simulink model "s_c856m1kfloat_sim"  (R2016b) (continued)
== system s_c856m1kfloat_sim == (continued)
      mask prompts: io_dir "I/O direction"; io_delay "I/O delay"; init_val "Initial Value"; sample_period "Sample period"; names "Bitfield names [msb...lsb]"; bitwidths "Bitfield widths"; bin_pts "Bitfield binary pts"; arith_types "Bitfield types, ufix=0, fix=1, bool=2"; sim_port "Provide sim input/output?"; show_format "Print format string?"
      mask help: A 32-bit software-accessible register. Can be divided into bitfields of varying widths and types using the fields in the block mask.
      mask init:
      | swreg_init(gcb)
      settings: AttributesFormatString=1output:uf32.0 = 32 bits
      mask TypeOptions io_dir: To Processor | From Processor
      mask Callback io_dir:
      | mask_enables = get_param(gcb, 'MaskEnables');
      | mask_names = get_param(gcb, 'MaskNames');
      | if strcmp(get_param(gcb, 'io_dir'), 'To Processor'),
      |     mask_enables{ismember(mask_names, 'sample_period')} = 'off';
      | else
      |     mask_enables{ismember(mask_names, 'sample_period')} = 'on';
      | end
      | set_param(gcb, 'MaskEnables', mask_enables);
      mask DialogControl ParameterTabVar0 Prompt: Setup
  wb_prog_delay : Subsystem  mask{n_bits_fft=fft_stages, n_bits_cd=17}
      mask prompts: n_bits_fft "fft length (2^?)"; n_bits_cd "max coarse delay (2^?)"
  wb_prog_delay1 : Subsystem  mask{n_bits_fft=fft_stages, n_bits_cd=17}
      mask prompts: n_bits_fft "fft length (2^?)"; n_bits_cd "max coarse delay (2^?)"
  we_delay112 : Subsystem  AncestorBlock=casper_library_delays/pipeline  mask(pipeline){latency=2}
      mask help:
      | An explicitly laid-out delay line to avoid mapping to SRL16s
      | for use in pipelining to help achieve timing closure.
      mask init:
      | pipeline_init(gcb,'latency',latency);
      settings: AttributesFormatString=Latency = %<latency>
      mask Display:
      | port_label('input', 1, 'd');
      | port_label('output', 1, 'q');
      | disp(['Z^-', num2str(latency)]);
  we_delay113 : Subsystem  AncestorBlock=casper_library_delays/pipeline  mask(pipeline){latency=2}
      mask help:
      | An explicitly laid-out delay line to avoid mapping to SRL16s
      | for use in pipelining to help achieve timing closure.
      mask init:
      | pipeline_init(gcb,'latency',latency);
      settings: AttributesFormatString=Latency = %<latency>
      mask Display:
      | port_label('input', 1, 'd');
      | port_label('output', 1, 'q');
      | disp(['Z^-', num2str(latency)]);
  we_delay114 : Subsystem  AncestorBlock=casper_library_delays/pipeline  mask(pipeline){latency=2}
      mask help:
      | An explicitly laid-out delay line to avoid mapping to SRL16s
      | for use in pipelining to help achieve timing closure.
      mask init:
      | pipeline_init(gcb,'latency',latency);
      settings: AttributesFormatString=Latency = %<latency>
      mask Display:
      | port_label('input', 1, 'd');
      | port_label('output', 1, 'q');
      | disp(['Z^-', num2str(latency)]);
  we_delay115 : Subsystem  AncestorBlock=casper_library_delays/pipeline  mask(pipeline){latency=2}
      mask help:
      | An explicitly laid-out delay line to avoid mapping to SRL16s
      | for use in pipelining to help achieve timing closure.
      mask init:
      | pipeline_init(gcb,'latency',latency);
      settings: AttributesFormatString=Latency = %<latency>
      mask Display:
      | port_label('input', 1, 'd');
      | port_label('output', 1, 'q');
      | disp(['Z^-', num2str(latency)]);
  we_delay116 : Subsystem  AncestorBlock=casper_library_delays/pipeline  mask(pipeline){latency=2}
      mask help:
      | An explicitly laid-out delay line to avoid mapping to SRL16s
      | for use in pipelining to help achieve timing closure.
      mask init:
      | pipeline_init(gcb,'latency',latency);
      settings: AttributesFormatString=Latency = %<latency>
      mask Display:
      | port_label('input', 1, 'd');
      | port_label('output', 1, 'q');
      | disp(['Z^-', num2str(latency)]);
  we_delay117 : Subsystem  AncestorBlock=casper_library_delays/pipeline  mask(pipeline){latency=2}
      mask help:
      | An explicitly laid-out delay line to avoid mapping to SRL16s
      | for use in pipelining to help achieve timing closure.
      mask init:
      | pipeline_init(gcb,'latency',latency);
      settings: AttributesFormatString=Latency = %<latency>
      mask Display:
      | port_label('input', 1, 'd');
      | port_label('output', 1, 'q');
      | disp(['Z^-', num2str(latency)]);
  we_delay124 : Subsystem  AncestorBlock=casper_library_delays/pipeline  mask(pipeline){latency=2}
      mask help:
      | An explicitly laid-out delay line to avoid mapping to SRL16s
      | for use in pipelining to help achieve timing closure.
      mask init:
      | pipeline_init(gcb,'latency',latency);
      settings: AttributesFormatString=Latency = %<latency>
      mask Display:
      | port_label('input', 1, 'd');
      | port_label('output', 1, 'q');
      | disp(['Z^-', num2str(latency)]);
  we_delay126 : Subsystem  AncestorBlock=casper_library_delays/pipeline  mask(pipeline){latency=2}
      mask help:
      | An explicitly laid-out delay line to avoid mapping to SRL16s
      | for use in pipelining to help achieve timing closure.
      mask init:
      | pipeline_init(gcb,'latency',latency);
      settings: AttributesFormatString=Latency = %<latency>
      mask Display:
      | port_label('input', 1, 'd');
      | port_label('output', 1, 'q');
      | disp(['Z^-', num2str(latency)]);
  we_delay13 : Subsystem  AncestorBlock=casper_library_delays/pipeline  mask(pipeline){latency=2}
      mask help:
      | An explicitly laid-out delay line to avoid mapping to SRL16s
      | for use in pipelining to help achieve timing closure.
      mask init:
      | pipeline_init(gcb,'latency',latency);
      settings: AttributesFormatString=Latency = %<latency>
      mask Display:
      | port_label('input', 1, 'd');
      | port_label('output', 1, 'q');
      | disp(['Z^-', num2str(latency)]);
  we_delay37 : Subsystem  AncestorBlock=casper_library_delays/pipeline  mask(pipeline){latency=2}
      mask help:
      | An explicitly laid-out delay line to avoid mapping to SRL16s
      | for use in pipelining to help achieve timing closure.
      mask init:
      | pipeline_init(gcb,'latency',latency);
      settings: AttributesFormatString=Latency = %<latency>
      mask Display:
      | port_label('input', 1, 'd');
      | port_label('output', 1, 'q');
      | disp(['Z^-', num2str(latency)]);
  we_delay38 : Subsystem  AncestorBlock=casper_library_delays/pipeline  mask(pipeline){latency=2}
      mask help:
      | An explicitly laid-out delay line to avoid mapping to SRL16s
      | for use in pipelining to help achieve timing closure.
      mask init:
      | pipeline_init(gcb,'latency',latency);
      settings: AttributesFormatString=Latency = %<latency>
      mask Display:
      | port_label('input', 1, 'd');
      | port_label('output', 1, 'q');
      | disp(['Z^-', num2str(latency)]);
  we_delay39 : Subsystem  AncestorBlock=casper_library_delays/pipeline  mask(pipeline){latency=2}
      mask help:
      | An explicitly laid-out delay line to avoid mapping to SRL16s
      | for use in pipelining to help achieve timing closure.
      mask init:
      | pipeline_init(gcb,'latency',latency);
      settings: AttributesFormatString=Latency = %<latency>
      mask Display:
      | port_label('input', 1, 'd');
      | port_label('output', 1, 'q');
      | disp(['Z^-', num2str(latency)]);
  we_delay41 : Subsystem  AncestorBlock=casper_library_delays/pipeline  mask(pipeline){latency=2}
      mask help:
      | An explicitly laid-out delay line to avoid mapping to SRL16s
      | for use in pipelining to help achieve timing closure.
      mask init:
      | pipeline_init(gcb,'latency',latency);
      settings: AttributesFormatString=Latency = %<latency>
      mask Display:
      | port_label('input', 1, 'd');
      | port_label('output', 1, 'q');
      | disp(['Z^-', num2str(latency)]);
  we_delay47 : Subsystem  AncestorBlock=casper_library_delays/pipeline  mask(pipeline){latency=2}
      mask help:
      | An explicitly laid-out delay line to avoid mapping to SRL16s
      | for use in pipelining to help achieve timing closure.
      mask init:
      | pipeline_init(gcb,'latency',latency);
      settings: AttributesFormatString=Latency = %<latency>
      mask Display:
      | port_label('input', 1, 'd');
      | port_label('output', 1, 'q');
      | disp(['Z^-', num2str(latency)]);
  we_delay48 : Subsystem  AncestorBlock=casper_library_delays/pipeline  mask(pipeline){latency=2}
      mask help:
      | An explicitly laid-out delay line to avoid mapping to SRL16s
      | for use in pipelining to help achieve timing closure.
      mask init:
      | pipeline_init(gcb,'latency',latency);
      settings: AttributesFormatString=Latency = %<latency>
      mask Display:
      | port_label('input', 1, 'd');
      | port_label('output', 1, 'q');
      | disp(['Z^-', num2str(latency)]);
  we_delay49 : Subsystem  AncestorBlock=casper_library_delays/pipeline  mask(pipeline){latency=2}
      mask help:
      | An explicitly laid-out delay line to avoid mapping to SRL16s
      | for use in pipelining to help achieve timing closure.
      mask init:
      | pipeline_init(gcb,'latency',latency);
      settings: AttributesFormatString=Latency = %<latency>
      mask Display:
      | port_label('input', 1, 'd');
      | port_label('output', 1, 'q');
      | disp(['Z^-', num2str(latency)]);
  we_delay50 : Subsystem  AncestorBlock=casper_library_delays/pipeline  mask(pipeline){latency=2}
      mask help:
      | An explicitly laid-out delay line to avoid mapping to SRL16s
      | for use in pipelining to help achieve timing closure.
      mask init:
      | pipeline_init(gcb,'latency',latency);
      settings: AttributesFormatString=Latency = %<latency>
      mask Display:
      | port_label('input', 1, 'd');
      | port_label('output', 1, 'q');
      | disp(['Z^-', num2str(latency)]);
  wng0 : Subsystem  mask{seed1=hex2dec('12345678'), seed2=hex2dec('87654321'), seed3=hex2dec('45612378'), seed4=hex2dec('85274163')}
      mask prompts: seed1 "Seed 1"; seed2 "Seed 2"; seed3 "Seed 3"; seed4 "Seed 4"
  wng1 : Subsystem  mask{seed1=hex2dec('65498732'), seed2=hex2dec('32198754'), seed3=hex2dec('45612378'), seed4=hex2dec('74563218')}
      mask prompts: seed1 "Seed 1"; seed2 "Seed 2"; seed3 "Seed 3"; seed4 "Seed 4"
  wng_corr : Subsystem  mask{seed1=hex2dec('75386429'), seed2=hex2dec('95123754'), seed3=hex2dec('98321495'), seed4=hex2dec('96478521')}
      mask prompts: seed1 "Seed 1"; seed2 "Seed 2"; seed3 "Seed 3"; seed4 "Seed 4"
  x_setup : Subsystem  Tag=xps:sw_reg  AncestorBlock=xps_library/software_register  mask(swreg){io_dir=From Processor, io_delay=0, init_val=0, sample_period=1, names=[f_per_x ip_per_x num_x snap_arm pktstart_mux pack_mux pktoffset_sel], bitwidths=[15 4 9 1 1 1 1], bin_pts=[0 2 0 0 0 0 0], arith_types=[0 0 0 2 0 2 0], sim_port=off, show_format=on}
      mask prompts: io_dir "I/O direction"; io_delay "I/O delay"; init_val "Initial Value"; sample_period "Sample period"; names "Bitfield names [msb...lsb]"; bitwidths "Bitfield widths"; bin_pts "Bitfield binary pts"; arith_types "Bitfield types, ufix=0, fix=1, bool=2"; sim_port "Provide sim input/output?"; show_format "Print format string?"
      mask help: A 32-bit software-accessible register. Can be divided into bitfields of varying widths and types using the fields in the block mask.
      mask init:
      | swreg_init(gcb)
      settings: AttributesFormatString=7 inputs:uf15.0,uf4.2,uf9.0,b,uf1.0,b,uf1.0 = 32 bits
      mask TypeOptions io_dir: To Processor | From Processor
      mask Callback io_dir:
      | mask_enables = get_param(gcb, 'MaskEnables');
      | mask_names = get_param(gcb, 'MaskNames');
      | if strcmp(get_param(gcb, 'io_dir'), 'To Processor'),
      |     mask_enables{ismember(mask_names, 'sample_period')} = 'off';
      | else
      |     mask_enables{ismember(mask_names, 'sample_period')} = 'on';
      | end
      | set_param(gcb, 'MaskEnables', mask_enables);
      mask DialogControl ParameterTabVar0 Prompt: Setup
  xsg_core_config : Subsystem  Tag=xps:xsg  AncestorBlock=xps_library/XSG_core_config  mask(xsg core config){hw_sys=SKARAB:xc7vx690t, clk_src=sys_clk, clk_rate=238, sample_period=1, synthesis_tool=XST}
      mask prompts: hw_sys "Hardware Platform"; clk_src "User IP Clock source"; clk_rate "User IP Clock Rate (MHz)"; sample_period "Sample Period"; synthesis_tool "Synthesis Tool:"
      mask help:
      | The XSG Core Config block is used to configure the System Generator design
      | for the casper_xps toolflow. Settings here are used to configure the
      | Xilinx System Generator block parameters automatically, and control toolflow
      | script  execution. It needs to be at the top level of all designs being compiled
      | with the casper_xps toolflow.
      mask init:
      | xps_xsg_conf_mask;
      mask Help: eval('xlWeb([getenv(''MLIB_ROOT''), ''\doc\html\sys_xsg_core_config\sys_xsg_core_config.html''])')
      mask TypeOptions hw_sys: ROACH:sx95t | ROACH:lx110t | ROACH2:sx475t | SNAP:xc7k160t | KC705:xc7k325t | SKARAB:xc7vx690t
      mask Callback hw_sys: xps_xsg_conf_callback
      mask TypeOptions clk_src: sys_clk | aux_clk
      mask Callback clk_src: xps_xsg_conf_callback
      mask Callback clk_rate: xps_xsg_conf_callback
      mask TypeOptions synthesis_tool: Leonardo Spectrum | Synplify | Synplify Pro | XST
      mask Display: fprintf(['MSSGE\n', hw_sys]);
  tag scope: cnt_rst=global, ct_board_hmc_offset=global, ct_chans_per_board=global, ct_chans_per_x=global, ct_chans_per_x_hmc_par=global, ct_chans_per_x_hmc_par_recip=global, ct_chans_per_x_par=global, ct_chans_per_x_recip=global, ct_fake_data_error=global, ct_freq_gen_offset=global, ct_num_x_boards=global, ct_num_x_boards_recip=global, ct_num_xeng=global, ct_num_xeng_recip=global, ct_rst_err_latch=global, ct_tag_insert_sel=global, ct_tvg_en2=global, ct_tvg_en=global, ct_x_per_board=global, ct_x_per_board_recip=global, force_gbe_txen=global, local_time_capture=global, local_time_src=global, obuf_read_gap=global, pfb_bypass=global, pktoffset_sel=global, pktstart_sel=global, reo_time_change=global, snap_reord_mux_we_sel=global, snap_stop_mux_sel=global, sys_rst=global, ts_tvg_en=global, tvgtone_levels=global, tvgtone_p0=global, tvgtone_p1=global, up_timestep_error=global
  wires:
  Constant3/1 -> gbe0/8(rx_overrun_ack)
  Constant4/1 -> gbe0/7(rx_ack)
  [gbe_rst] -> pipeline99/1(d)
  unpack_and_reorder/4(recvd) -> ts_tvg/5(recvd_in)
  sync_gen/2(dout) -> bus_expand6/1(bus_in)
  sync_gen/3(dvalid) -> impulse_gen1/6(en), impulse_gen0/6(en)
  Slice12/1 -> [time80]
  impulse_gen0/3(dvalid) -> Gateway Out125/1, [valid80]
  impulse_gen0/1(sync_out) -> Gateway Out124/1, [sync80]
  impulse_gen0/2(dout) -> [d80_adc0]
  impulse_gen0/4(misco) -> [time80_48], Slice12/1
  [tvg_adc0_en] -> impulse_gen0/1(tvg_en)
  impulse_gen1/1(sync_out) -> Terminator6/1
  impulse_gen1/2(dout) -> [d80_adc1]
  impulse_gen1/3(dvalid) -> Terminator8/1
  impulse_gen1/4(misco) -> [dig_flags]
  [tvg_adc1_en] -> impulse_gen1/1(tvg_en)
  impulse0/1(in_offset) -> Slice7/1
  impulse0/2(in_amplitude) -> pipeline205/1(d)
  impulse1/1(in_offset) -> Slice8/1
  impulse1/2(in_amplitude) -> pipeline207/1(d)
  sync_gen/1(sync) -> impulse_gen0/4(sync_in), impulse_gen1/4(sync_in)
  bus_expand6/1(msb_out2) -> impulse_gen0/5(din)
  bus_expand6/2(lsb_out1) -> impulse_gen1/5(din)
  sync_gen/5(misco) -> impulse_gen1/7(misci)
  sync_gen/4(time_out) -> impulse_gen0/7(misci)
  Constant31/1 -> Concat9/2
  [unpack_timestamp] -> Concat9/1
  [up_timestep_error] -> Logical15/1
  Concat9/1 -> sync_gen/1(time_in)
  [sys_relock] -> sync_gen/5(relock)
  [unpack_dv] -> sync_gen/2(en)
  [unpack_d160] -> sync_gen/3(din)
  Slice7/1 -> pipeline204/1(d)
  Slice8/1 -> pipeline206/1(d)
  trig_time_msw/1(in_msw) -> pipeline28/1(d)
  trig_time_lsw/1(in_lsw) -> pipeline44/1(d)
  edge_detect4/1(out) -> pipeline132/1(d)
  control/7(in_cnt_rst) -> edge_detect1/1(in)
  edge_detect1/1(out) -> pipeline66/1(d)
  control/3(in_gbe_rst) -> pipeline131/1(d)
  control/1(in_sys_rst) -> pipeline146/1(d)
  pipeline146/1(q) -> [sys_rst]
  pipeline132/1(q) -> [clr_status]
  pipeline131/1(q) -> [gbe_rst]
  pipeline128/1(q) -> [gbe_txen]
  pipeline127/1(q) -> [tvg_adc0_en]
  pipeline66/1(q) -> [cnt_rst]
  control/5(in_tvg_adc0) -> pipeline127/1(d)
  edge_detect2/1(out) -> pipeline59/1(d)
  pipeline59/1(q) -> [gbe_cnt_rst]
  control/8(in_gbe_cnt_rst) -> edge_detect2/1(in)
  control/4(in_gbe_txen) -> pipeline128/1(d)
  control/2(in_status_clr) -> edge_detect4/1(in)
  pipeline52/1(q) -> [adc_snap_arm]
  control/9(in_adc_snap_arm) -> pipeline52/1(d)
  control/10(in_adc_snap_trig_select) -> pipeline47/1(d)
  pipeline47/1(q) -> [adc_snap_trig_source]
  fft_shift/1(in_fft_shift) -> pipeline4/1(d)
  pfb/5(of) -> [pfb_of]
  pfb/4(dvalid) -> [pfb_dv]
  pfb/1(sync_out) -> [pfb_sync]
  [gbe_cnt_rst] -> pipeline31/1(d)
  pipeline31/1(q) -> gbe0/9(debug_rst)
  gbe0/13(rx_dest_ip) -> pipeline115/1(d)
  gbe0/14(rx_dest_port) -> pipeline116/1(d)
  [rx_dest_ip] -> pipeline/1(d)
  pipeline/1(q) -> rx_dest_ip/1(out_reg)
  gbe0/1(led_up) -> pipeline100/1(d)
  [forty_gbe_led_up] -> pipeline19/1(d)
  gbe0/2(led_rx) -> pipeline101/1(d)
  [forty_gbe_led_rx] -> pipeline20/1(d)
  gbe0/3(led_tx) -> pipeline102/1(d)
  [forty_gbe_led_tx] -> pipeline51/1(d)
  gbe0/4(tx_afull) -> pipeline103/1(d)
  gbe0/5(tx_overflow) -> pipeline104/1(d)
  gbe0/12(rx_overrun) -> pipeline114/1(d)
  pipeline19/1(q) -> gbe_status/1(out_led_up)
  pipeline20/1(q) -> gbe_status/2(out_led_rx)
  pipeline51/1(q) -> gbe_status/3(out_led_tx)
  gbe0/8(rx_source_ip) -> pipeline110/1(d)
  gbe0/9(rx_source_port) -> pipeline111/1(d)
  gbe0/6(rx_data) -> pipeline108/1(d)
  gbe0/10(rx_end_of_frame) -> pipeline112/1(d)
  gbe0/11(rx_bad_frame) -> pipeline113/1(d)
  [s256_d] -> pipeline98/1(d)
  [s256_ip] -> pipeline97/1(d)
  [s256_port] -> pipeline96/1(d)
  Logical2/1 -> gbe0/6(tx_end_of_frame)
  [s256_v] -> Logical1/2
  [s256_eof] -> Logical2/2
  Delay1/1 -> Logical1/1, Logical2/1
  [s256_eof] -> Delay1/2
  pipeline96/1(q) -> gbe0/5(tx_dest_port)
  pipeline97/1(q) -> gbe0/4(tx_dest_ip)
  pipeline98/1(q) -> gbe0/2(tx_data)
  pipeline99/1(q) -> gbe0/1(rst)
  Logical4/1 -> Delay1/1
  pipeline100/1(q) -> [forty_gbe_led_up]
  pipeline101/1(q) -> [forty_gbe_led_rx]
  pipeline102/1(q) -> [forty_gbe_led_tx]
  pipeline103/1(q) -> [forty_gbe_tx_full]
  pipeline104/1(q) -> [forty_gbe_tx_of]
  pipeline108/1(q) -> [forty_gbe_rx_data]
  pipeline110/1(q) -> [forty_gbe_rx_src_ip]
  pipeline111/1(q) -> [forty_gbe_rx_src_port]
  pipeline112/1(q) -> [forty_gbe_rx_eof]
  pipeline113/1(q) -> [forty_gbe_rx_bad]
  pipeline114/1(q) -> [forty_gbe_rx_overrun]
  pipeline115/1(q) -> [rx_dest_ip]
  pipeline116/1(q) -> [rx_dest_port]
  [forty_gbe_rx_data] -> unpack_and_reorder/1(d256)
  [forty_gbe_rx_valid] -> unpack_and_reorder/2(dv256)
  [forty_gbe_rx_eof] -> unpack_and_reorder/3(eof256)
  unpack_and_reorder/1(d160) -> ts_tvg/2(data_in)
  unpack_and_reorder/2(dv) -> ts_tvg/3(dv_in)
  unpack_and_reorder/3(time) -> ts_tvg/4(time_in)
  pipeline11/1(q) -> [unpack_d160]
  pipeline12/1(q) -> [unpack_dv]
  pipeline15/1(q) -> [unpack_timestamp]
  Constant1/1 -> sync_gen/4(misci)
  pipeline4/1(q) -> [fft_shift]
  Logical1/1 -> gbe0/3(tx_valid)
  pipeline43/1(q) -> [ct_tvg_en]
  control/11(in_ct_tvg_en) -> pipeline43/1(d)
  pipeline39/1(q) -> [up_dbg_rst]
  control/12(in_unpack_debug_reset) -> pipeline39/1(d)
  [clr_status] -> Subsystem3/1(rst)
  Slice/1 -> Subsystem3/2(in0)
  [pfb_of] -> Slice/1, Slice6/1
  Slice6/1 -> Subsystem3/3(in1)
  [pfb_sync] -> Subsystem3/4(in2)
  Subsystem3/3(out2) -> pipeline213/1(d)
  Subsystem3/1(out0) -> pipeline211/1(d)
  Subsystem3/2(out1) -> pipeline212/1(d)
  [delay_sync] -> pipeline208/1(d)
  [delay_dv] -> pipeline228/1(d)
  [delay_d0] -> pipeline229/1(d)
  [delay_d1] -> pipeline230/1(d)
  control/13(in_cd_bypass) -> pipeline38/1(d)
  pipeline38/1(q) -> [cd_bypass]
  Concat22/1 -> [ld_val0]
  Concat3/1 -> [ld_val1]
  phase0/1(in_initial) -> Reinterpret2/1
  delta_delay0/1(in_delta) -> Reinterpret1/1
  phase0/2(in_delta) -> Reinterpret3/1
  Reinterpret8/1 -> pipeline126/1(d)
  Reinterpret1/1 -> pipeline123/1(d)
  Reinterpret2/1 -> pipeline119/1(d)
  Reinterpret3/1 -> pipeline57/1(d)
  phase1/1(in_initial) -> Reinterpret5/1
  delta_delay1/1(in_delta) -> Reinterpret4/1
  phase1/2(in_delta) -> Reinterpret6/1
  Reinterpret4/1 -> pipeline49/1(d)
  Reinterpret5/1 -> pipeline46/1(d)
  Reinterpret6/1 -> pipeline32/1(d)
  Reinterpret7/1 -> pipeline50/1(d)
  delay0/1(in_initial) -> Reinterpret8/1
  delay1/1(in_initial) -> Reinterpret7/1
  x_setup/1(in_f_per_x) -> pipeline107/1(d)
  x_setup/2(in_ip_per_x) -> pipeline120/1(d)
  x_setup/3(in_num_x) -> pipeline121/1(d)
  x_setup/4(in_snap_arm) -> pipeline124/1(d)
  x_setup/5(in_pktstart_mux) -> pipeline125/1(d)
  x_setup/6(in_pack_mux) -> pipeline130/1(d)
  tl_cd0_control0/3(in_load_time_msw) -> Concat6/1
  tl_cd0_control/1(in_load_time_lsw) -> Concat6/2
  Constant42/1 -> tl_cd0_control/1(sim_1)
  Constant40/1 -> tl_cd0_control0/3(sim_3)
  Constant41/1 -> tl_cd0_control0/2(sim_2)
  Constant43/1 -> Delay12/1
  Delay12/1 -> tl_cd0_control0/1(sim_1)
  Concat6/1 -> pipeline136/1(d)
  tl_cd0_control0/2(in_load_immediate) -> pipeline137/1(d)
  tl_cd0_control0/1(in_arm) -> pipeline138/1(d)
  tl_cd1_control0/3(in_load_time_msw) -> Concat8/1
  tl_cd1_control/1(in_load_time_lsw) -> Concat8/2
  Constant47/1 -> tl_cd1_control/1(sim_1)
  Constant45/1 -> tl_cd1_control0/3(sim_3)
  Constant46/1 -> tl_cd1_control0/2(sim_2)
  Constant48/1 -> Delay13/1
  Delay13/1 -> tl_cd1_control0/1(sim_1)
  Concat8/1 -> pipeline143/1(d)
  tl_cd1_control0/2(in_load_immediate) -> pipeline134/1(d)
  tl_cd1_control0/1(in_arm) -> pipeline135/1(d)
  [ld_val0] -> pipeline73/1(d)
  [clr_status] -> pipeline25/1(d), pipeline75/1(d)
  tl_cd0/6(time) -> [time80_tl_cd0]
  Constant6/1 -> Concat15/2
  Concat15/1 -> pipeline24/1(d), Gateway Out7/1, pipeline74/1(d)
  [time80] -> Concat15/1
  pipeline75/1(q) -> tl_cd0/1(rst)
  pipeline74/1(q) -> tl_cd0/2(current_time)
  pipeline73/1(q) -> tl_cd0/3(load_value)
  pipeline72/1(q) -> Gateway Out128/1, tl_cd0/4(sync_in)
  pipeline71/1(q) -> tl_cd0/5(din)
  pipeline76/1(q) -> Gateway Out126/1, tl_cd0/6(en)
  Constant16/1 -> tl_cd0/7(misci)
  tl_cd0/7(misco) -> Terminator/1
  tl_cd0/3(sync_out) -> Gateway Out104/1, [sync_tl_cd0]
  tl_cd0/5(dvalid) -> Gateway Out127/1, [dvalid_tl_cd0]
  tl_cd0/4(dout) -> [dout_tl_cd0]
  tl_cd0/2(enable) -> [en_tl_cd0]
  tl_cd0/1(value) -> [delay_tl_cd0]
  pipeline92/1(q) -> tl_cd0/10(load_time)
  pipeline91/1(q) -> tl_cd0/9(load_immediate)
  pipeline90/1(q) -> tl_cd0/8(arm)
  [tl_cd0_arm] -> pipeline90/1(d)
  [tl_cd0_load_immediate] -> pipeline91/1(d)
  [tl_cd0_load_time] -> pipeline92/1(d)
  Gateway Out7/1 -> To Workspace7/1
  [dvalid_tl_cd0] -> Delay22/1
  [sync_tl_cd0] -> Delay21/1
  [en_tl_cd0] -> Delay24/1
  [delay_tl_cd0] -> Delay23/1
  Concat5/1 -> time_gen_cd/6(rd_misc_in)
  time_gen_cd/4(rd_misc_out) -> bus_expand2/1(bus_in)
  [sync_tl_cd0] -> time_gen_cd/1(wr_sync_in)
  [dvalid_tl_cd0] -> time_gen_cd/2(wr_en)
  [hmc_sync_p0] -> time_gen_cd/4(rd_sync_in)
  [hmc_dvalid] -> time_gen_cd/5(rd_en)
  time_gen_cd/1(rd_sync_out) -> [tg_rd_sync]
  time_gen_cd/2(rd_dvalid) -> [tg_rd_dvalid]
  [dvalid_tl_bs0] -> wb_prog_delay/4(en)
  [delay_tl_bs0] -> wb_prog_delay/2(delay_val)
  [delay_tl_sync0] -> wb_prog_delay/1(sync)
  [en_tl_bs0] -> wb_prog_delay/5(load_val)
  wb_prog_delay/2(data_out) -> [delay_d0]
  wb_prog_delay/3(dvalid) -> [delay_dv]
  [time80_tl_cd0] -> Slice9/1
  Slice9/1 -> time_gen_cd/3(wr_val_in)
  Constant35/1 -> Concat7/2
  time_gen_cd/3(rd_val_out) -> Concat7/1
  Concat7/1 -> [tg_rd_time_out]
  wb_prog_delay/1(sync_out) -> Gateway Out107/1, [delay_sync]
  pipeline156/1(q) -> tl_cd_bs0/1(rst)
  pipeline89/1(q) -> tl_cd_bs0/2(current_time)
  pipeline88/1(q) -> tl_cd_bs0/3(load_value)
  pipeline84/1(q) -> tl_cd_bs0/4(sync_in)
  pipeline78/1(q) -> tl_cd_bs0/5(din)
  pipeline77/1(q) -> tl_cd_bs0/6(en)
  [clr_status] -> pipeline156/1(d)
  [tg_rd_time_out] -> pipeline89/1(d)
  [ld_val0] -> pipeline88/1(d)
  [tg_rd_sync] -> pipeline84/1(d)
  [tg_rd_dvalid] -> pipeline77/1(d)
  Constant34/1 -> tl_cd_bs0/7(misci)
  tl_cd_bs0/7(misco) -> Terminator12/1
  tl_cd_bs0/4(dout) -> [data_tl_bs0]
  tl_cd_bs0/6(time) -> Terminator13/1
  tl_cd_bs0/5(dvalid) -> [dvalid_tl_bs0]
  tl_cd_bs0/1(value) -> [delay_tl_bs0]
  tl_cd_bs0/3(sync_out) -> Gateway Out106/1, [delay_tl_sync0]
  tl_cd_bs0/2(enable) -> [en_tl_bs0]
  pipeline69/1(q) -> tl_cd_bs0/8(arm)
  pipeline68/1(q) -> tl_cd_bs0/9(load_immediate)
  pipeline67/1(q) -> tl_cd_bs0/10(load_time)
  [tl_cd0_arm] -> pipeline69/1(d)
  [tl_cd0_load_immediate] -> pipeline68/1(d)
  [tl_cd0_load_time] -> pipeline67/1(d)
  [data_tl_bs0] -> wb_prog_delay/3(din)
  [tg_data_p0] -> pipeline78/1(d)
  pipeline25/1(q) -> tl_cd1/1(rst)
  [ld_val1] -> pipeline26/1(d)
  pipeline24/1(q) -> tl_cd1/2(current_time)
  pipeline26/1(q) -> tl_cd1/3(load_value)
  pipeline36/1(q) -> tl_cd1/4(sync_in)
  pipeline33/1(q) -> tl_cd1/5(din)
  pipeline34/1(q) -> tl_cd1/6(en)
  pipeline37/1(q) -> tl_cd1/9(load_immediate)
  pipeline35/1(q) -> tl_cd1/8(arm)
  pipeline40/1(q) -> tl_cd1/10(load_time)
  [tl_cd1_arm] -> pipeline35/1(d)
  [tl_cd1_load_immediate] -> pipeline37/1(d)
  [tl_cd1_load_time] -> pipeline40/1(d)
  Constant17/1 -> tl_cd1/7(misci)
  tl_cd1/1(value) -> [delay_tl_cd1]
  tl_cd1/2(enable) -> [en_tl_cd1]
  tl_cd1/3(sync_out) -> [sync_tl_cd1]
  tl_cd1/4(dout) -> [dout_tl_cd1]
  tl_cd1/5(dvalid) -> [dvalid_tl_cd1]
  tl_cd1/6(time) -> [time80_tl_cd1]
  tl_cd1/7(misco) -> Terminator1/1
  [delay_tl_cd1] -> Delay27/1
  [en_tl_cd1] -> Delay28/1
  [sync_tl_cd1] -> Delay25/1
  [dvalid_tl_cd1] -> Delay26/1
  pipeline61/1(q) -> tl_cd_bs1/1(rst)
  pipeline60/1(q) -> tl_cd_bs1/2(current_time)
  pipeline54/1(q) -> tl_cd_bs1/3(load_value)
  pipeline53/1(q) -> tl_cd_bs1/4(sync_in)
  pipeline23/1(q) -> tl_cd_bs1/5(din)
  pipeline22/1(q) -> tl_cd_bs1/6(en)
  [clr_status] -> pipeline61/1(d)
  [tg_rd_time_out] -> pipeline60/1(d)
  [ld_val1] -> pipeline54/1(d)
  [tg_rd_sync] -> pipeline53/1(d)
  [tg_rd_dvalid] -> pipeline22/1(d)
  [tg_data_p1] -> pipeline23/1(d)
  Constant36/1 -> tl_cd_bs1/7(misci)
  pipeline21/1(q) -> tl_cd_bs1/8(arm)
  pipeline1/1(q) -> tl_cd_bs1/9(load_immediate)
  pipeline70/1(q) -> tl_cd_bs1/10(load_time)
  [tl_cd1_load_time] -> pipeline70/1(d)
  [tl_cd1_load_immediate] -> pipeline1/1(d)
  [tl_cd1_arm] -> pipeline21/1(d)
  tl_cd_bs1/7(misco) -> Terminator10/1
  tl_cd_bs1/4(dout) -> [data_tl_bs1]
  tl_cd_bs1/6(time) -> Terminator7/1
  tl_cd_bs1/5(dvalid) -> [dvalid_tl_bs1]
  tl_cd_bs1/1(value) -> [delay_tl_bs1]
  tl_cd_bs1/3(sync_out) -> [delay_tl_sync1]
  tl_cd_bs1/2(enable) -> [en_tl_bs1]
  [delay_tl_sync1] -> wb_prog_delay1/1(sync)
  [delay_tl_bs1] -> wb_prog_delay1/2(delay_val)
  [en_tl_bs1] -> wb_prog_delay1/5(load_val)
  [data_tl_bs1] -> wb_prog_delay1/3(din)
  [dvalid_tl_bs1] -> wb_prog_delay1/4(en)
  wb_prog_delay1/2(data_out) -> [delay_d1]
  wb_prog_delay1/1(sync_out) -> Terminator16/1
  wb_prog_delay1/3(dvalid) -> Terminator11/1
  Subsystem7/1(per_sec) -> pipeline192/1(d)
  [upg_reo_time] -> Subsystem1/3(time)
  [upg_reo_recv] -> Subsystem1/4(recv)
  [upg_reo_dv] -> Subsystem7/1(dv), Subsystem1/2(dv)
  [cnt_rst] -> Subsystem1/1(rst)
  Subsystem1/7(time_change) -> [reo_time_change]
  Logical5/1 -> Subsystem1/7(overflow_err)
  [upg_of80_p1] -> Logical5/2
  [upg_of80_p0] -> Logical5/1
  Subsystem1/8(timestep_err) -> [up_timestep_error]
  [upg_reo_disc] -> Subsystem1/5(discard_err)
  [upg_reo_relock] -> Subsystem1/6(relock_err)
  Subsystem1/1(timestep_err_cnt) -> pipeline189/1(d)
  Subsystem1/2(recv_err_cnt) -> pipeline188/1(d)
  Subsystem1/3(discard_err_cnt) -> pipeline164/1(d)
  Subsystem1/4(relock_err_cnt) -> pipeline163/1(d)
  Subsystem1/5(overflow_err_cnt) -> pipeline190/1(d)
  Subsystem1/6(dv_cnt) -> pipeline191/1(d)
  pipeline93/1(q) -> [tvg_adc1_en]
  control/6(in_tvg_adc1) -> pipeline93/1(d)
  gbe0/7(rx_valid) -> pipeline62/1(d)
  pipeline62/1(q) -> [forty_gbe_rx_valid]
  Counter1/1 -> pipeline165/1(d)
  pipeline165/1(q) -> sync80_count/1(out_reg)
  pipeline65/1(q) -> Counter1/1
  pipeline109/1(q) -> Counter1/2
  [cnt_rst] -> edge_detect9/1(in)
  [sync80] -> edge_detect10/1(in)
  edge_detect9/1(out) -> pipeline65/1(d)
  edge_detect10/1(out) -> pipeline109/1(d)
  Convert/1 -> [f_per_x]
  [hmc_data_p0] -> Concat5/1
  [hmc_data_p1] -> Concat5/2
  bus_expand2/1(msb_out2) -> [tg_data_p0]
  bus_expand2/2(lsb_out1) -> [tg_data_p1]
  control/14(in_gbe_stopmux) -> pipeline14/1(d)
  pipeline14/1(q) -> [gbe_stopmux]
  x_setup/7(in_pktoffset_sel) -> pipeline149/1(d)
  control/15(in_pfb_bypass) -> pipeline13/1(d)
  pipeline13/1(q) -> [pfb_bypass]
  Delay21/1 -> cd_hmc/1(sync_in)
  Delay22/1 -> cd_hmc/3(en)
  Delay23/1 -> cd_hmc/4(delay_val)
  Delay24/1 -> cd_hmc/5(load_val)
  [dout_tl_cd0] -> Delay29/1
  pipeline138/1(q) -> [tl_cd0_arm]
  pipeline137/1(q) -> [tl_cd0_load_immediate]
  pipeline136/1(q) -> [tl_cd0_load_time]
  pipeline134/1(q) -> [tl_cd1_load_immediate]
  pipeline135/1(q) -> [tl_cd1_arm]
  pipeline143/1(q) -> [tl_cd1_load_time]
  Delay29/1 -> cd_hmc/2(din)
  [dout_tl_cd1] -> Delay30/1
  ts_tvg/1(d160) -> pipeline11/1(d)
  ts_tvg/2(dv) -> pipeline12/1(d)
  ts_tvg/3(time) -> pipeline15/1(d)
  ts_tvg/4(recvd) -> Terminator9/1
  control/16(in_ts_tvg_en) -> pipeline10/1(d)
  pipeline10/1(q) -> [ts_tvg_en]
  [ts_tvg_en] -> ts_tvg/1(tvg_en)
  [pfbin_d0] -> pfb/3(din0)
  [fft_shift] -> pfb/1(shift)
  [pfbin_sync] -> pfb/2(sync_in)
  [pfbin_d1] -> pfb/4(din1)
  [pfbin_dv] -> pfb/5(en)
  Delay25/1 -> cd_hmc/6(sync_in1)
  Delay30/1 -> cd_hmc/7(din1)
  Delay26/1 -> cd_hmc/8(en1)
  Delay27/1 -> cd_hmc/9(delay_val1)
  Delay28/1 -> cd_hmc/10(load_val1)
  iptx_base/1(in_reg) -> pipeline105/1(d)
  pipeline105/1(q) -> [tx_ip_base]
  tx_metadata/1(in_porttx) -> pipeline63/1(d)
  tx_metadata/2(in_board_id) -> pipeline106/1(d)
  tvg_tone_levels/2(in_p0i) -> bus_create/2(in2)
  tvg_tone_levels/3(in_p1r) -> bus_create/3(in3)
  tvg_tone_levels/4(in_p1i) -> bus_create/4(in4)
  tvg_tone_levels/1(in_p0r) -> bus_create/1(in1)
  tvg_tone_targets/1(in_p0) -> Slice18/1
  tvg_tone_targets/2(in_p1) -> Slice19/1
  Constant51/1 -> tvg_tone_targets/1(sim_1)
  Constant52/1 -> tvg_tone_targets/2(sim_2)
  Constant37/1 -> tvg_tone_levels/4(sim_4)
  Constant2/1 -> tvg_tone_levels/1(sim_1)
  Constant27/1 -> tvg_tone_levels/3(sim_3)
  Constant5/1 -> tvg_tone_levels/2(sim_2)
  bus_create/1(bus_out) -> [tvgtone_levels]
  pipeline17/1(q) -> [outfreq_stopmux]
  ct_debug/6(in_outfreq_stopmux) -> pipeline17/1(d)
  pipeline18/1(q) -> [out_stopmux]
  ct_debug/5(in_out_stopmux) -> pipeline18/1(d)
  pipeline55/1(q) -> [wrrd_stopmux]
  ct_debug/3(in_wrrd_stopmux) -> pipeline55/1(d)
  pipeline56/1(q) -> [in_stopmux]
  ct_debug/1(in_in_stopmux) -> pipeline56/1(d)
  pipeline122/1(q) -> [ct_snap_arm]
  pipeline147/1(q) -> edge_detect5/1
  ct_debug/8(in_snap_trig) -> pipeline147/1(d)
  ct_debug/7(in_snap_arm) -> pipeline122/1(d)
  edge_detect5/1 -> [ct_snap_trig]
  ct_debug/2(in_in_trigmux) -> pipeline150/1(d)
  pipeline150/1(q) -> [in_trigmux]
  ct_debug/4(in_obuf_stopmux) -> pipeline167/1(d)
  pipeline167/1(q) -> [obuf_stopmux]
  ct_debug/9(in_snap_wesel) -> pipeline8/1(d)
  pipeline8/1(q) -> [ct_snap_wesel]
  pipeline157/1(q) -> [ct_snap_trigsel]
  ct_debug/10(in_snap_trigsel) -> pipeline157/1(d)
  pipeline166/1(q) -> [ct_snap_circsel]
  ct_debug/11(in_snap_circsel) -> pipeline166/1(d)
  we_delay49/1(q) -> [ct_tag_insert_sel]
  edge_detect3/1 -> [ct_rst_err_latch]
  we_delay47/1(q) -> edge_detect3/1
  we_delay48/1(q) -> [ct_fake_data_error]
  we_delay13/1(q) -> [ct_tvg_en]
  we_delay50/1(q) -> [ct_tvg_en2]
  Constant7/1 -> ct_control0/5(sim_5)
  Constant21/1 -> ct_control0/4(sim_4)
  Constant20/1 -> ct_control0/3(sim_3)
  Constant22/1 -> ct_control0/2(sim_2)
  Constant15/1 -> ct_control0/1(sim_1)
  ct_control0/1(in_tvg_en) -> we_delay13/1(d)
  ct_control0/2(in_tvg_en2) -> we_delay50/1(d)
  ct_control0/3(in_rst_err_latch) -> we_delay47/1(d)
  ct_control0/4(in_fake_error) -> we_delay48/1(d)
  ct_control0/5(in_tag_insert) -> we_delay49/1(d)
  we_delay126/1(q) -> [obuf_read_gap]
  ct_control0/6(in_obuf_read_gap) -> we_delay126/1(d)
  Constant30/1 -> ct_control0/6(sim_6)
  we_delay41/1(q) -> [ct_num_xeng]
  we_delay112/1(q) -> [ct_num_xeng_recip]  "NUM_X_RECIP"
  ct_control1/2(in_num_x_recip) -> we_delay112/1(d)
  ct_control1/1(in_num_x) -> we_delay41/1(d)
  we_delay114/1(q) -> [ct_x_per_board]
  ct_control1/3(in_x_per_board) -> we_delay114/1(d)
  we_delay39/1(q) -> [ct_chans_per_x], Slice3/1  "PER_X"
  we_delay113/1(q) -> [ct_chans_per_board]
  ct_control2/1(in_chans_per_x) -> we_delay39/1(d)
  ct_control2/2(in_chans_per_board) -> we_delay113/1(d)
  Slice3/1 -> Slice39/1, [ct_chans_per_x_par]  "PARPAR"
  we_delay115/1(q) -> [ct_x_per_board_recip]
  ct_control1/4(in_x_per_board_recip) -> we_delay115/1(d)
  we_delay38/1(q) -> [ct_num_x_boards]
  ct_control3/1(in_num_x_boards) -> we_delay38/1(d)
  we_delay37/1(q) -> [ct_num_x_boards_recip]
  ct_control3/2(in_num_x_boards_recip) -> we_delay37/1(d)
  ct_control3/3(in_chans_per_x_recip) -> we_delay124/1(d)
  we_delay124/1(q) -> Reinterpret40/1, [ct_chans_per_x_recip]
  Slice39/1 -> [ct_chans_per_x_hmc_par]  "per_x_hmc"
  Reinterpret40/1 -> [ct_chans_per_x_hmc_par_recip]
  Constant49/1 -> ct_control1/1(sim_1)
  Constant53/1 -> ct_control1/2(sim_2)
  Constant33/1 -> ct_control1/3(sim_3)
  Constant23/1 -> ct_control2/1(sim_1)
  Constant50/1 -> ct_control2/2(sim_2)
  Constant54/1 -> ct_control1/4(sim_4)
  Constant32/1 -> ct_control3/1(sim_1)
  Constant14/1 -> ct_control3/2(sim_2)
  Constant44/1 -> ct_control3/3(sim_3)
  Slice18/1 -> [tvgtone_p0]
  Slice19/1 -> [tvgtone_p1]
  [hmc_dvalid] -> Subsystem26/1(In1)
  Subsystem26/1(Out1) -> pipeline209/1(d)
  [dvalid_tl_cd0] -> Subsystem25/1(In1)
  Subsystem25/1(Out1) -> pipeline210/1(d)
  Slice36/1 -> we_delay116/1(d)
  Constant38/1 -> ct_control4/1(sim_1)
  ct_control4/1(in_ct_board_offset) -> Slice36/1
  ct_control5/1(in_ct_freq_gen_offset) -> we_delay117/1(d)
  Constant39/1 -> ct_control5/1(sim_1)
  we_delay116/1(q) -> [ct_board_hmc_offset]
  we_delay117/1(q) -> [ct_freq_gen_offset]
  sync_mangle/1(sync_out) -> [hmc_sync_p0]
  sync_mangle/2(dout0) -> [hmc_data_p0]
  sync_mangle/4(dvalid) -> [hmc_dvalid]
  cd_hmc/1(sync_out) -> Delay8/1, sync_mangle/1(sync_in)
  cd_hmc/2(dout0) -> sync_mangle/2(din)
  cd_hmc/4(dvalid) -> sync_mangle/4(en)
  cd_hmc/5(hmc_error) -> [cd_error]
  cd_hmc/3(dout1) -> sync_mangle/3(din1)
  sync_mangle/3(dout1) -> [hmc_data_p1]
  control/17(in_force_gbe_txen) -> pipeline9/1(d)
  pipeline9/1(q) -> [force_gbe_txen]
  [gbe_txen] -> Logical4/2
  [force_gbe_txen] -> Logical6/2
  Logical6/1 -> Logical4/1
  [upg_sperr_magic] -> Subsystem33/2(in0)
  [upg_sperr_len] -> Subsystem33/5(in3)
  [upg_sperr_hdr] -> Subsystem33/3(in1)
  [upg_sperr_pad] -> Subsystem33/4(in2)
  Subsystem33/5(out4) -> pipeline155/1(d)
  [upg_sperr_time] -> Subsystem33/6(in4)
  Subsystem33/4(out3) -> pipeline158/1(d)
  Subsystem33/3(out2) -> pipeline159/1(d)
  Subsystem33/2(out1) -> pipeline160/1(d)
  Subsystem33/1(out0) -> pipeline161/1(d)
  [cnt_rst] -> Subsystem4/1(rst), Subsystem33/1(rst)
  Logical3/1 -> Subsystem4/2(in0)
  Subsystem4/1(out0) -> pipeline162/1(d)
  [upg_sp_dv] -> Logical3/2
  [upg_sp_eof] -> Logical3/1
  control/18(in_snap_stop_mux_sel) -> pipeline7/1(d)
  control/19(in_snap_reord_mux_we_sel) -> pipeline6/1(d)
  pipeline7/1(q) -> [snap_stop_mux_sel]
  pipeline6/1(q) -> [snap_reord_mux_we_sel]
  Delay8/1 -> Gateway Out105/1, [hmc_sync_raw]
  control/20(in_local_time_source) -> pipeline5/1(d)
  edge_detect6/1(out) -> pipeline3/1(d)
  pipeline3/1(q) -> [local_time_capture]
  pipeline5/1(q) -> [local_time_src]
  control/21(in_local_time_capture) -> edge_detect6/1(in)
  unpack_and_reorder/5(spead_timestamp) -> [spead_timestamp]
  control/22(in_time_diff_check_en) -> pipeline2/1(d)
  pipeline2/1(q) -> [time_diff_check_en]
  Logical15/1 -> Logical7/1
  Logical7/1 -> Logical8/2
  [sys_rst] -> Logical8/1
  Logical8/1 -> edge_detect17/1(in)
  [time_diff_error] -> Logical15/4
  [cd_error] -> Logical15/2
  [ct_error] -> Logical15/3
  sync_gen/6(waiting) -> [sync_armed]
  [synced] -> posedge_delay/1(in)
  control/23(in_auto_rst_enable) -> pipeline148/1(d)
  pipeline148/1(q) -> [auto_rst_enable]
  posedge_delay/1(out) -> Logical10/2
  [auto_rst_enable] -> Delay47/1
  Logical10/1 -> [auto_rst_armed], Logical7/2
  edge_detect17/1(out) -> [sys_relock], Register/2
  [s256_sync] -> Register/3, Register/1
  Register/1 -> [synced]
  [synced] -> Logical6/1
  Delay47/1 -> Logical10/1
  float_to_fix/1(Out1) -> pipeline145/1(d)
  float_to_fix1/1(Out1) -> pipeline144/1(d)
  pfb/2(dout0) -> [pfb_dfloat_0]
  pfb/3(dout1) -> [pfb_dfloat_1]
  [pfb_dfloat_0] -> pipeline234/1(d)
  [pfb_dfloat_1] -> pipeline231/1(d)
  [scale_float] -> pipeline233/1(d)
  [scale_float] -> pipeline232/1(d)
  pipeline144/1(q) -> [pfb_d144_1]
  pipeline145/1(q) -> [pfb_d144_0]
  pipeline28/1(q) -> Terminator14/1
  pipeline44/1(q) -> Terminator15/1
  pipeline30/1(q) -> [scale_float]
  scale_float/1(in_scale) -> pipeline30/1(d)
  pipeline126/1(q) -> Concat22/1
  pipeline123/1(q) -> Concat22/2
  pipeline119/1(q) -> Concat22/3
  pipeline57/1(q) -> Concat22/4
  pipeline50/1(q) -> Concat3/1
  pipeline49/1(q) -> Concat3/2
  pipeline46/1(q) -> Concat3/3
  pipeline32/1(q) -> Concat3/4
  pipeline161/1(q) -> unpack_status/1(out_magic_err_cnt)
  pipeline160/1(q) -> unpack_status/2(out_header_err_cnt)
  pipeline159/1(q) -> unpack_status/3(out_pad_err_cnt)
  pipeline158/1(q) -> unpack_status/4(out_pkt_len_err_cnt)
  pipeline155/1(q) -> unpack_status/5(out_time_err_cnt)
  pipeline162/1(q) -> unpack_status1/1(out_pkt_cnt)
  pipeline189/1(q) -> reorder_status/1(out_timestep_err_cnt)
  pipeline188/1(q) -> reorder_status/2(out_receive_err_cnt)
  pipeline164/1(q) -> reorder_status/3(out_discard_cnt)
  pipeline163/1(q) -> reorder_status/4(out_relock_err_cnt)
  pipeline190/1(q) -> reorder_status/5(out_overflow_err_cnt)
  pipeline191/1(q) -> reorder_status/6(out_dv_cnt)
  pipeline192/1(q) -> reorder_status1/1(out_dv_per_sec)
  pipeline205/1(q) -> impulse_gen0/3(impulse_amplitude)
  pipeline204/1(q) -> impulse_gen0/2(impulse_offset)
  pipeline206/1(q) -> impulse_gen1/2(impulse_offset)
  pipeline207/1(q) -> impulse_gen1/3(impulse_amplitude)
  pipeline209/1(q) -> cd_out_dv_rate/1(out_reg)
  pipeline210/1(q) -> cd_in_dv_rate/1(out_reg)
  pipeline211/1(q) -> pfb_status/1(out_pol0_or_err_cnt)
  pipeline212/1(q) -> pfb_status/2(out_pol1_or_err_cnt)
  pipeline213/1(q) -> pfb_status/3(out_sync_cnt)
  pipeline63/1(q) -> [tx_port]
  pipeline106/1(q) -> [tx_board_id]
  pipeline107/1(q) -> Convert/1
  pipeline120/1(q) -> [ip_per_x]
  pipeline121/1(q) -> [num_x]
  pipeline124/1(q) -> [pack_snap_arm]
  pipeline125/1(q) -> [pktstart_sel]
  pipeline130/1(q) -> [pack_mux]
  pipeline149/1(q) -> [pktoffset_sel]
  pipeline208/1(q) -> [pfbin_sync]
  pipeline228/1(q) -> [pfbin_dv]
  pipeline229/1(q) -> [pfbin_d0]
  pipeline230/1(q) -> [pfbin_d1]
  pipeline231/1(q) -> float_to_fix1/1(dfloat_in)
  pipeline232/1(q) -> float_to_fix1/2(scale)
  pipeline233/1(q) -> float_to_fix/2(scale)
  pipeline234/1(q) -> float_to_fix/1(dfloat_in)
  cwg_corr/1(bus_out) -> pipeline257/1(d)
  bus_addsub2/1(dout) -> Delay35/1
  bus_addsub4/1(dout) -> bus_convert6/1(din)
  bus_mult/1(a*b) -> [cwg0_tp1], bus_convert/1(din)
  bus_addsub5/1(dout) -> bus_convert7/1(din)
  bus_mult1/1(a*b) -> bus_convert1/1(din), [cwg1_tp1]
  scale_out1/1(in_scale) -> Gateway Out3/1, pipeline254/1(d)
  Delay35/1 -> bus_convert4/1(din)
  Delay139/1 -> bus_addsub4/1(a)
  Delay36/1 -> bus_addsub5/1(a)
  Delay86/1 -> bus_addsub5/2(b)
  pipeline255/1(q) -> bus_mult1/1(a), [cwg1_tp0]
  pipeline254/1(q) -> bus_mult1/2(b)
  pipeline253/1(q) -> bus_mult/2(b)
  [cwg_corr] -> bus_addsub2/2(b)
  pipeline236/1(q) -> [cwg_80_0]
  pipeline237/1(q) -> [cwg_80_1]
  Delay33/1 -> bus_addsub4/2(b)
  bus_convert/1(dout) -> [cwg0_tp2], pipeline236/1(d)
  bus_convert4/1(dout) -> [cwg_corr_out0]
  bus_convert5/1(dout) -> [cwg_corr_out1]
  bus_convert6/1(dout) -> pipeline252/1(d)
  bus_convert7/1(dout) -> pipeline255/1(d)
  scale_out0/1(in_scale) -> Gateway Out5/1, pipeline253/1(d)
  Constant64/1 -> freq_cwg0/1(sim_1)
  scale_cwg0/1(in_scale) -> pipeline247/1(d)
  freq_cwg0/1(in_frequency) -> pipeline248/1(d)
  Constant79/1 -> freq_cwg_corr/1(sim_1)
  freq_cwg_corr/1(in_frequency) -> pipeline250/1(d)
  scale_cwg_corr/1(in_scale) -> pipeline249/1(d)
  Constant80/1 -> scale_cwg_corr/1(sim_1)
  Constant60/1 -> scale_out1/1(sim_1)
  Constant59/1 -> scale_out0/1(sim_1)
  pipeline248/1(q) -> cwg0/1(freq)
  pipeline247/1(q) -> cwg0/2(scale)
  Constant74/1 -> pipeline240/1(d)
  Constant62/1 -> rpt_length_cwg0/1(sim_1)
  rpt_en_cwg0/1(in_en) -> Convert25/1
  Constant70/1 -> rpt_en_cwg0/1(sim_1)
  Convert25/1 -> pipeline241/1(d)
  cwg0/1(bus_out) -> pipeline258/1(d)
  rpt_length_cwg0/1(in_repeat_length) -> pipeline242/1(d)
  pipeline240/1(q) -> cwg0/3(rst)
  pipeline241/1(q) -> cwg0/4(repeat_en)
  pipeline242/1(q) -> cwg0/5(repeat_length)
  cwg0/3(rpt_rst) -> Terminator61/1
  pipeline250/1(q) -> cwg_corr/1(freq)
  pipeline249/1(q) -> cwg_corr/2(scale)
  pipeline235/1(q) -> cwg_corr/3(rst)
  pipeline238/1(q) -> cwg_corr/4(repeat_en)
  pipeline239/1(q) -> cwg_corr/5(repeat_length)
  Constant96/1 -> cwg_corr/6(en)
  Constant72/1 -> pipeline235/1(d)
  rpt_en_cwg_corr/1(in_en) -> pipeline238/1(d)
  cwg_corr/3(rpt_rst) -> Terminator64/1
  cwg_corr/2(dvalid) -> Terminator65/1
  Constant97/1 -> rpt_en_cwg_corr/1(sim_1)
  rpt_length_cwg_corr/1(in_repeat_length) -> pipeline239/1(d)
  Constant99/1 -> rpt_length_cwg_corr/1(sim_1)
  pipeline252/1(q) -> [cwg0_tp0], bus_mult/1(a)
  Gateway Out3/1 -> To Workspace3/1
  Gateway Out5/1 -> To Workspace5/1
  bus_convert1/1(dout) -> [cwg1_tp2], pipeline237/1(d)
  Constant106/1 -> cwg0/6(en)
  pipeline258/1(q) -> [cwg0], bus_expand1/1(bus_in)
  cwg0/2(dvalid) -> Terminator30/1
  [wng_sum0] -> Delay139/1
  [cwg_corr_out0] -> Delay33/1
  [cwg0] -> bus_addsub2/1(a)
  [cwg_corr_out1] -> Delay86/1
  Constant57/1 -> scale_cwg0/1(sim_1)
  Constant56/1 -> freq_cwg1/1(sim_1)
  scale_cwg1/1(in_scale) -> pipeline246/1(d)
  freq_cwg1/1(in_frequency) -> pipeline251/1(d)
  pipeline251/1(q) -> cwg1/1(freq)
  pipeline246/1(q) -> cwg1/2(scale)
  Constant73/1 -> pipeline243/1(d)
  Constant55/1 -> rpt_length_cwg1/1(sim_1)
  rpt_en_cwg1/1(in_en) -> Convert2/1
  Constant58/1 -> rpt_en_cwg1/1(sim_1)
  Convert2/1 -> pipeline244/1(d)
  cwg1/1(bus_out) -> pipeline256/1(d)
  rpt_length_cwg1/1(in_repeat_length) -> pipeline245/1(d)
  pipeline243/1(q) -> cwg1/3(rst)
  pipeline244/1(q) -> cwg1/4(repeat_en)
  pipeline245/1(q) -> cwg1/5(repeat_length)
  cwg1/3(rpt_rst) -> Terminator29/1
  Constant9/1 -> cwg1/6(en)
  pipeline256/1(q) -> [cwg1]
  cwg1/2(dvalid) -> Terminator28/1
  Constant12/1 -> scale_cwg1/1(sim_1)
  pipeline257/1(q) -> [cwg_corr]
  [cwg1] -> bus_addsub3/1(a)
  bus_addsub3/1(dout) -> Delay32/1
  Delay32/1 -> bus_convert5/1(din)
  [cwg_corr] -> bus_addsub3/2(b)
  [wng_sum1] -> Delay36/1
  Gateway Out31/1 -> To Workspace30/1
  Gateway Out28/1 -> To Workspace21/1
  Gateway Out29/1 -> To Workspace29/1
  Gateway Out30/1 -> To Workspace28/1
  bus_expand3/1(msb_out8) -> Gateway Out28/1
  bus_expand3/2(out7) -> Gateway Out31/1
  bus_expand3/4(out5) -> Gateway Out30/1
  bus_expand3/3(out6) -> Gateway Out29/1
  bus_expand3/8(lsb_out1) -> Gateway Out34/1
  bus_expand3/7(out2) -> Gateway Out33/1
  bus_expand3/6(out3) -> Gateway Out35/1
  bus_expand3/5(out4) -> Gateway Out32/1
  Gateway Out34/1 -> To Workspace20/1
  Gateway Out33/1 -> To Workspace22/1
  Gateway Out35/1 -> To Workspace23/1
  Gateway Out32/1 -> To Workspace27/1
  [cwg_80_0] -> bus_expand3/1(bus_in)
  Gateway Out39/1 -> To Workspace37/1
  Gateway Out36/1 -> To Workspace31/1
  Gateway Out37/1 -> To Workspace36/1
  Gateway Out38/1 -> To Workspace35/1
  bus_expand4/1(msb_out8) -> Gateway Out36/1
  bus_expand4/2(out7) -> Gateway Out39/1
  bus_expand4/4(out5) -> Gateway Out38/1
  bus_expand4/3(out6) -> Gateway Out37/1
  bus_expand4/8(lsb_out1) -> Gateway Out42/1
  bus_expand4/7(out2) -> Gateway Out41/1
  bus_expand4/6(out3) -> Gateway Out43/1
  bus_expand4/5(out4) -> Gateway Out40/1
  Gateway Out42/1 -> To Workspace19/1
  Gateway Out41/1 -> To Workspace32/1
  Gateway Out43/1 -> To Workspace33/1
  Gateway Out40/1 -> To Workspace34/1
  [cwg_80_1] -> bus_expand4/1(bus_in)
  [d80_adc0] -> Mux2/2
  [d80_adc1] -> Mux3/2
  [cwg_80_0] -> Mux2/3
  [cwg_80_1] -> Mux3/3
  [d80_adc1] -> Terminator17/1
  [d80_adc0] -> Terminator18/1
  Mux3/1 -> pipeline33/1(d)
  Mux2/1 -> pipeline71/1(d)
  Constant/1 -> Mux2/1
  Constant61/1 -> Mux3/1
  scale_wng1/1(in_scale) -> Gateway Out6/1, pipeline264/1(d)
  scale_wng_corr/1(in_scale) -> pipeline263/1(d)
  [wng0_tp0] -> pipeline265/1(d)
  [wng1_tp0] -> pipeline268/1(d)
  Constant63/1 -> scale_wng_corr/1(sim_1)
  pipeline262/1(q) -> [wng_scale0]
  pipeline263/1(q) -> [wng_scale_corr]
  pipeline264/1(q) -> [wng_scale1]
  pipeline265/1(q) -> bus_addsub/1(a)
  pipeline266/1(q) -> bus_addsub/2(b)
  pipeline267/1(q) -> bus_addsub1/1(a)
  [wng_corr_tp0] -> pipeline267/1(d), pipeline266/1(d)
  pipeline268/1(q) -> bus_addsub1/2(b)
  bus_convert2/1(dout) -> [wng_sum0]
  bus_convert3/1(dout) -> [wng_sum1]
  Constant66/1 -> scale_wng1/1(sim_1)
  scale_wng0/1(in_scale) -> Gateway Out17/1, pipeline262/1(d)
  Constant65/1 -> man_rst_wng0/1(sim_1)
  Logical20/1 -> pipeline261/1(d)
  man_rst_wng0/1(in_rst) -> [wng_man_rst0], Logical20/2
  Constant68/1 -> Logical20/1
  pipeline259/1(q) -> wng1/2(rst)
  Logical21/1 -> pipeline259/1(d)
  man_rst_wng1/1(in_rst) -> [wng_man_rst1], Logical21/2
  Constant69/1 -> Logical21/1
  Constant100/1 -> man_rst_wng1/1(sim_1)
  pipeline260/1(q) -> wng_corr/2(rst)
  Logical24/1 -> pipeline260/1(d)
  man_rst_wng_corr/1(in_rst) -> [wng_man_rst_corr], Logical24/2
  Constant71/1 -> Logical24/1
  Constant101/1 -> man_rst_wng_corr/1(sim_1)
  Gateway Out6/1 -> To Workspace6/1
  Gateway Out17/1 -> To Workspace17/1
  Constant67/1 -> scale_wng0/1(sim_1)
  bus_addsub1/1(dout) -> Delay37/1
  Delay37/1 -> bus_convert3/1(din)
  bus_addsub/1(dout) -> Delay40/1
  Delay40/1 -> bus_convert2/1(din)
  [wng_scale0] -> wng0/1(scale_16_14)
  pipeline261/1(q) -> wng0/2(rst)
  wng0/1(bus_out) -> [wng0_tp0]
  [wng_scale1] -> wng1/1(scale_16_14)
  [wng_scale_corr] -> wng_corr/1(scale_16_14)
  wng1/1(bus_out) -> [wng1_tp0]
  wng_corr/1(bus_out) -> [wng_corr_tp0]
  Gateway Out8/1 -> To Workspace41/1
  Gateway Out1/1 -> To Workspace51/1
  Gateway Out2/1 -> To Workspace40/1
  Gateway Out4/1 -> To Workspace39/1
  bus_expand5/1(msb_out8) -> Gateway Out1/1
  bus_expand5/2(out7) -> Gateway Out8/1
  bus_expand5/4(out5) -> Gateway Out4/1
  bus_expand5/3(out6) -> Gateway Out2/1
  bus_expand5/8(lsb_out1) -> Gateway Out11/1
  bus_expand5/7(out2) -> Gateway Out10/1
  bus_expand5/6(out3) -> Gateway Out12/1
  bus_expand5/5(out4) -> Gateway Out9/1
  Gateway Out11/1 -> To Workspace38/1
  Gateway Out10/1 -> To Workspace47/1
  Gateway Out12/1 -> To Workspace52/1
  Gateway Out9/1 -> To Workspace53/1
  [pfb_d144_0] -> bus_expand5/1(bus_in)
  Constant8/1 -> [s256_d]
  Constant10/1 -> [s256_v]
  Constant11/1 -> [s256_ip]
  Constant13/1 -> [s256_port]
  Constant18/1 -> [s256_eof]
  Constant19/1 -> [ct_error]
  Constant24/1 -> [time_diff_error]
  Constant25/1 -> [s256_sync]
  Constant26/1 -> scale_float/1(sim_1)
  Gateway Out84/1 -> To Workspace12/1
  Gateway Out70/1 -> To Workspace4/1
  Gateway Out80/1 -> To Workspace11/1
  Gateway Out83/1 -> To Workspace10/1
  bus_expand12/1(msb_out8) -> Reinterpret9/1
  bus_expand12/2(out7) -> Reinterpret10/1
  bus_expand12/4(out5) -> Reinterpret12/1
  bus_expand12/3(out6) -> Reinterpret11/1
  bus_expand12/8(lsb_out1) -> Reinterpret15/1
  bus_expand12/7(out2) -> Reinterpret14/1
  bus_expand12/6(out3) -> Reinterpret13/1
  bus_expand12/5(out4) -> Reinterpret16/1
  Gateway Out72/1 -> To Workspace1/1
  Gateway Out71/1 -> To Workspace2/1
  Gateway Out73/1 -> To Workspace8/1
  Gateway Out85/1 -> To Workspace9/1
  Gateway Out81/1 -> To Workspace25/1
  Gateway Out78/1 -> To Workspace18/1
  Gateway Out79/1 -> To Workspace16/1
  bus_expand13/1(msb_out8) -> Reinterpret20/1
  bus_expand13/2(out7) -> Reinterpret17/1
  bus_expand13/4(out5) -> Reinterpret19/1
  bus_expand13/3(out6) -> Reinterpret18/1
  bus_expand13/8(lsb_out1) -> Reinterpret23/1
  bus_expand13/7(out2) -> Reinterpret22/1
  bus_expand13/6(out3) -> Reinterpret21/1
  bus_expand13/5(out4) -> Reinterpret24/1
  Gateway Out76/1 -> To Workspace13/1
  Gateway Out75/1 -> To Workspace24/1
  Gateway Out77/1 -> To Workspace14/1
  Gateway Out82/1 -> To Workspace15/1
  Gateway Out74/1 -> To Workspace26/1
  [pfb_dfloat_0] -> bus_expand12/1(bus_in)
  [pfb_dfloat_1] -> bus_expand13/1(bus_in)
  Reinterpret9/1 -> Gateway Out70/1
  Reinterpret10/1 -> Gateway Out84/1
  Reinterpret11/1 -> Gateway Out80/1
  Reinterpret12/1 -> Gateway Out83/1
  Reinterpret13/1 -> Gateway Out73/1
  Reinterpret15/1 -> Gateway Out72/1
  Reinterpret14/1 -> Gateway Out71/1
  Reinterpret16/1 -> Gateway Out85/1
  Reinterpret19/1 -> Gateway Out79/1
  Reinterpret20/1 -> Gateway Out74/1
  Reinterpret17/1 -> Gateway Out81/1
  Reinterpret18/1 -> Gateway Out78/1
  Reinterpret21/1 -> Gateway Out77/1
  Reinterpret24/1 -> Gateway Out82/1
  Reinterpret23/1 -> Gateway Out76/1
  Reinterpret22/1 -> Gateway Out75/1
  Gateway Out86/1 -> To Workspace86/1
  [pfb_sync] -> Gateway Out86/1
  Gateway Out87/1 -> To Workspace87/1
  [delay_sync] -> Gateway Out87/1
  Gateway Out104/1 -> To Workspace104/1
  Gateway Out105/1 -> To Workspace105/1
  Gateway Out106/1 -> To Workspace106/1
  Gateway Out107/1 -> To Workspace107/1
  Gateway Out124/1 -> To Workspace124/1
  Gateway Out125/1 -> To Workspace125/1
  Gateway Out126/1 -> To Workspace126/1
  Gateway Out127/1 -> To Workspace127/1
  [valid80] -> Terminator2/1
  Constant28/1 -> pipeline34/1(d), pipeline76/1(d)
  [sync80] -> Terminator3/1
  Constant29/1 -> edge_detect7/1(in)
  edge_detect7/1(out) -> Delay2/1
  Delay2/1 -> pipeline36/1(d), pipeline72/1(d)
  Gateway Out128/1 -> To Workspace128/1
  [pfb_sync] -> pipeline16/1(d)
  Gateway Out129/1 -> To Workspace129/1
  Delay/1 -> pipeline27/1(d)
  pipeline16/1(q) -> Delay/1
  pipeline27/1(q) -> Gateway Out129/1
